simulink model "bee2_calib_corr"  (Simulink 7.1)
settings: Solver=ode45  StartTime=0.0  StopTime=100  RelTol=1e-3
undefined symbols (not defined in the file or in its folder): BEE2_usr
libraries: xbsIndex_r4 x2291, quant_xcorr_lib x1792, xps_library x56, xbsReplacements_r4 x32, casper_library_misc x2
(part 15 of 30)

== system bee2_calib_corr/quant_cross_corr1/lag-4 ==
  in1_0 : Inport  OutDataType=sfix(16)  OutScaling=2^0
  in1_1 : Inport  Port=2  OutDataType=sfix(16)  OutScaling=2^0
  in1_2 : Inport  Port=3  OutDataType=sfix(16)  OutScaling=2^0
  in1_3 : Inport  Port=4  OutDataType=sfix(16)  OutScaling=2^0
  in1_4 : Inport  Port=5  OutDataType=sfix(16)  OutScaling=2^0
  in1_5 : Inport  Port=6  OutDataType=sfix(16)  OutScaling=2^0
  in1_6 : Inport  Port=7  OutDataType=sfix(16)  OutScaling=2^0
  in1_7 : Inport  Port=8  OutDataType=sfix(16)  OutScaling=2^0
  in2_0 : Inport  Port=9  OutDataType=sfix(16)  OutScaling=2^0
  in2_1 : Inport  Port=10  OutDataType=sfix(16)  OutScaling=2^0
  in2_2 : Inport  Port=11  OutDataType=sfix(16)  OutScaling=2^0
  in2_3 : Inport  Port=12  OutDataType=sfix(16)  OutScaling=2^0
  in2_4 : Inport  Port=13  OutDataType=sfix(16)  OutScaling=2^0
  in2_5 : Inport  Port=14  OutDataType=sfix(16)  OutScaling=2^0
  in2_6 : Inport  Port=15  OutDataType=sfix(16)  OutScaling=2^0
  in2_7 : Inport  Port=16  OutDataType=sfix(16)  OutScaling=2^0
  rst : Inport  Port=17  OutDataType=sfix(16)  OutScaling=2^0
  en : Inport  Port=18  OutDataType=sfix(16)  OutScaling=2^0
  accumul-4 : xbsIndex_r4/Accumulator  operation=Add  n_bits=32  overflow=Saturate  scale=1  rst=on  hasbypass=off  en=on  dbl_ovrd=off  use_behavioral_HDL=on  implementation=Fabric  block_type=accum
      settings: infoedit="Adder or subtractor-based accumulator.   Output type and binary point position match the input.<P><P>Hardware notes: When \"Reinitialize with input 'b' on reset\" is selected, the accumulator is forced to run at the system rate even if the input 'b' is running at a slower rate."  xl_area=[0, 0, 0, 0, 0, 0, 0]  block_version=10.1.3
  add1_{1..4} (4 alike) : xbsIndex_r4/AddSub  mode=Addition  use_carryin=off  use_carryout=off  en=off  latency=1  precision=Full  arith_type=Unsigned  n_bits=8  bin_pt=2  quantization=Truncate  overflow=Wrap  dbl_ovrd=off  use_behavioral_HDL=on  pipelined=on  use_rpm=on  hw_selection=Fabric  block_type=addsub
      settings: xl_area=[0, 0, 0, 0, 0, 0, 0]  block_version=10.1.3
  add2_1 : xbsIndex_r4/AddSub  mode=Addition  use_carryin=off  use_carryout=off  en=off  latency=1  precision=Full  arith_type=Unsigned  n_bits=8  bin_pt=2  quantization=Truncate  overflow=Wrap  dbl_ovrd=off  use_behavioral_HDL=on  pipelined=on  use_rpm=on  hw_selection=Fabric  block_type=addsub
      settings: xl_area=[0, 0, 0, 0, 0, 0, 0]  block_version=10.1.3
  add2_2 : xbsIndex_r4/AddSub  mode=Addition  use_carryin=off  use_carryout=off  en=off  latency=1  precision=Full  arith_type=Unsigned  n_bits=8  bin_pt=2  quantization=Truncate  overflow=Wrap  dbl_ovrd=off  use_behavioral_HDL=on  pipelined=on  use_rpm=on  hw_selection=Fabric  block_type=addsub
      settings: xl_area=[0, 0, 0, 0, 0, 0, 0]  block_version=10.1.3
  add3_1 : xbsIndex_r4/AddSub  mode=Addition  use_carryin=off  use_carryout=off  en=off  latency=1  precision=Full  arith_type=Unsigned  n_bits=8  bin_pt=2  quantization=Truncate  overflow=Wrap  dbl_ovrd=off  use_behavioral_HDL=on  pipelined=on  use_rpm=on  hw_selection=Fabric  block_type=addsub
      settings: xl_area=[0, 0, 0, 0, 0, 0, 0]  block_version=10.1.3
  mult{1..8} (8 alike) : quant_xcorr_lib/2bit_mult  SystemSampleTime=-1
  acc_out : Outport  OutDataType=sfix(16)  OutScaling=2^0
  wires:
  in1_0/1 -> mult1/1
  in2_0/1 -> mult1/2
  in1_1/1 -> mult2/1
  in2_1/1 -> mult2/2
  in1_2/1 -> mult3/1
  in2_2/1 -> mult3/2
  in1_3/1 -> mult4/1
  in2_3/1 -> mult4/2
  in1_4/1 -> mult5/1
  in2_4/1 -> mult5/2
  in1_5/1 -> mult6/1
  in2_5/1 -> mult6/2
  in1_6/1 -> mult7/1
  in2_6/1 -> mult7/2
  in1_7/1 -> mult8/1
  in2_7/1 -> mult8/2
  mult1/1 -> add1_1/1
  mult2/1 -> add1_1/2
  mult3/1 -> add1_2/1
  mult4/1 -> add1_2/2
  mult5/1 -> add1_3/1
  mult6/1 -> add1_3/2
  mult7/1 -> add1_4/1
  mult8/1 -> add1_4/2
  add1_1/1 -> add2_1/1
  add1_2/1 -> add2_1/2
  add1_3/1 -> add2_2/1
  add1_4/1 -> add2_2/2
  add2_1/1 -> add3_1/1
  add2_2/1 -> add3_1/2
  add3_1/1 -> accumul-4/1
  accumul-4/1 -> acc_out/1
  rst/1 -> accumul-4/2
  en/1 -> accumul-4/3

== system bee2_calib_corr/quant_cross_corr1/lag-5 ==
  in1_0 : Inport  OutDataType=sfix(16)  OutScaling=2^0
  in1_1 : Inport  Port=2  OutDataType=sfix(16)  OutScaling=2^0
  in1_2 : Inport  Port=3  OutDataType=sfix(16)  OutScaling=2^0
  in1_3 : Inport  Port=4  OutDataType=sfix(16)  OutScaling=2^0
  in1_4 : Inport  Port=5  OutDataType=sfix(16)  OutScaling=2^0
  in1_5 : Inport  Port=6  OutDataType=sfix(16)  OutScaling=2^0
  in1_6 : Inport  Port=7  OutDataType=sfix(16)  OutScaling=2^0
  in1_7 : Inport  Port=8  OutDataType=sfix(16)  OutScaling=2^0
  in2_0 : Inport  Port=9  OutDataType=sfix(16)  OutScaling=2^0
  in2_1 : Inport  Port=10  OutDataType=sfix(16)  OutScaling=2^0
  in2_2 : Inport  Port=11  OutDataType=sfix(16)  OutScaling=2^0
  in2_3 : Inport  Port=12  OutDataType=sfix(16)  OutScaling=2^0
  in2_4 : Inport  Port=13  OutDataType=sfix(16)  OutScaling=2^0
  in2_5 : Inport  Port=14  OutDataType=sfix(16)  OutScaling=2^0
  in2_6 : Inport  Port=15  OutDataType=sfix(16)  OutScaling=2^0
  in2_7 : Inport  Port=16  OutDataType=sfix(16)  OutScaling=2^0
  rst : Inport  Port=17  OutDataType=sfix(16)  OutScaling=2^0
  en : Inport  Port=18  OutDataType=sfix(16)  OutScaling=2^0
  accumul-5 : xbsIndex_r4/Accumulator  operation=Add  n_bits=32  overflow=Saturate  scale=1  rst=on  hasbypass=off  en=on  dbl_ovrd=off  use_behavioral_HDL=on  implementation=Fabric  block_type=accum
      settings: infoedit="Adder or subtractor-based accumulator.   Output type and binary point position match the input.<P><P>Hardware notes: When \"Reinitialize with input 'b' on reset\" is selected, the accumulator is forced to run at the system rate even if the input 'b' is running at a slower rate."  xl_area=[0, 0, 0, 0, 0, 0, 0]  block_version=10.1.3
  add1_{1..4} (4 alike) : xbsIndex_r4/AddSub  mode=Addition  use_carryin=off  use_carryout=off  en=off  latency=1  precision=Full  arith_type=Unsigned  n_bits=8  bin_pt=2  quantization=Truncate  overflow=Wrap  dbl_ovrd=off  use_behavioral_HDL=on  pipelined=on  use_rpm=on  hw_selection=Fabric  block_type=addsub
      settings: xl_area=[0, 0, 0, 0, 0, 0, 0]  block_version=10.1.3
  add2_1 : xbsIndex_r4/AddSub  mode=Addition  use_carryin=off  use_carryout=off  en=off  latency=1  precision=Full  arith_type=Unsigned  n_bits=8  bin_pt=2  quantization=Truncate  overflow=Wrap  dbl_ovrd=off  use_behavioral_HDL=on  pipelined=on  use_rpm=on  hw_selection=Fabric  block_type=addsub
      settings: xl_area=[0, 0, 0, 0, 0, 0, 0]  block_version=10.1.3
  add2_2 : xbsIndex_r4/AddSub  mode=Addition  use_carryin=off  use_carryout=off  en=off  latency=1  precision=Full  arith_type=Unsigned  n_bits=8  bin_pt=2  quantization=Truncate  overflow=Wrap  dbl_ovrd=off  use_behavioral_HDL=on  pipelined=on  use_rpm=on  hw_selection=Fabric  block_type=addsub
      settings: xl_area=[0, 0, 0, 0, 0, 0, 0]  block_version=10.1.3
  add3_1 : xbsIndex_r4/AddSub  mode=Addition  use_carryin=off  use_carryout=off  en=off  latency=1  precision=Full  arith_type=Unsigned  n_bits=8  bin_pt=2  quantization=Truncate  overflow=Wrap  dbl_ovrd=off  use_behavioral_HDL=on  pipelined=on  use_rpm=on  hw_selection=Fabric  block_type=addsub
      settings: xl_area=[0, 0, 0, 0, 0, 0, 0]  block_version=10.1.3
  mult{1..8} (8 alike) : quant_xcorr_lib/2bit_mult  SystemSampleTime=-1
  acc_out : Outport  OutDataType=sfix(16)  OutScaling=2^0
  wires:
  in1_0/1 -> mult1/1
  in2_0/1 -> mult1/2
  in1_1/1 -> mult2/1
  in2_1/1 -> mult2/2
  in1_2/1 -> mult3/1
  in2_2/1 -> mult3/2
  in1_3/1 -> mult4/1
  in2_3/1 -> mult4/2
  in1_4/1 -> mult5/1
  in2_4/1 -> mult5/2
  in1_5/1 -> mult6/1
  in2_5/1 -> mult6/2
  in1_6/1 -> mult7/1
  in2_6/1 -> mult7/2
  in1_7/1 -> mult8/1
  in2_7/1 -> mult8/2
  mult1/1 -> add1_1/1
  mult2/1 -> add1_1/2
  mult3/1 -> add1_2/1
  mult4/1 -> add1_2/2
  mult5/1 -> add1_3/1
  mult6/1 -> add1_3/2
  mult7/1 -> add1_4/1
  mult8/1 -> add1_4/2
  add1_1/1 -> add2_1/1
  add1_2/1 -> add2_1/2
  add1_3/1 -> add2_2/1
  add1_4/1 -> add2_2/2
  add2_1/1 -> add3_1/1
  add2_2/1 -> add3_1/2
  add3_1/1 -> accumul-5/1
  accumul-5/1 -> acc_out/1
  rst/1 -> accumul-5/2
  en/1 -> accumul-5/3

== system bee2_calib_corr/quant_cross_corr1/lag-6 ==
  in1_0 : Inport  OutDataType=sfix(16)  OutScaling=2^0
  in1_1 : Inport  Port=2  OutDataType=sfix(16)  OutScaling=2^0
  in1_2 : Inport  Port=3  OutDataType=sfix(16)  OutScaling=2^0
  in1_3 : Inport  Port=4  OutDataType=sfix(16)  OutScaling=2^0
  in1_4 : Inport  Port=5  OutDataType=sfix(16)  OutScaling=2^0
  in1_5 : Inport  Port=6  OutDataType=sfix(16)  OutScaling=2^0
  in1_6 : Inport  Port=7  OutDataType=sfix(16)  OutScaling=2^0
  in1_7 : Inport  Port=8  OutDataType=sfix(16)  OutScaling=2^0
  in2_0 : Inport  Port=9  OutDataType=sfix(16)  OutScaling=2^0
  in2_1 : Inport  Port=10  OutDataType=sfix(16)  OutScaling=2^0
  in2_2 : Inport  Port=11  OutDataType=sfix(16)  OutScaling=2^0
  in2_3 : Inport  Port=12  OutDataType=sfix(16)  OutScaling=2^0
  in2_4 : Inport  Port=13  OutDataType=sfix(16)  OutScaling=2^0
  in2_5 : Inport  Port=14  OutDataType=sfix(16)  OutScaling=2^0
  in2_6 : Inport  Port=15  OutDataType=sfix(16)  OutScaling=2^0
  in2_7 : Inport  Port=16  OutDataType=sfix(16)  OutScaling=2^0
  rst : Inport  Port=17  OutDataType=sfix(16)  OutScaling=2^0
  en : Inport  Port=18  OutDataType=sfix(16)  OutScaling=2^0
  accumul-6 : xbsIndex_r4/Accumulator  operation=Add  n_bits=32  overflow=Saturate  scale=1  rst=on  hasbypass=off  en=on  dbl_ovrd=off  use_behavioral_HDL=on  implementation=Fabric  block_type=accum
      settings: infoedit="Adder or subtractor-based accumulator.   Output type and binary point position match the input.<P><P>Hardware notes: When \"Reinitialize with input 'b' on reset\" is selected, the accumulator is forced to run at the system rate even if the input 'b' is running at a slower rate."  xl_area=[0, 0, 0, 0, 0, 0, 0]  block_version=10.1.3
  add1_{1..4} (4 alike) : xbsIndex_r4/AddSub  mode=Addition  use_carryin=off  use_carryout=off  en=off  latency=1  precision=Full  arith_type=Unsigned  n_bits=8  bin_pt=2  quantization=Truncate  overflow=Wrap  dbl_ovrd=off  use_behavioral_HDL=on  pipelined=on  use_rpm=on  hw_selection=Fabric  block_type=addsub
      settings: xl_area=[0, 0, 0, 0, 0, 0, 0]  block_version=10.1.3
  add2_1 : xbsIndex_r4/AddSub  mode=Addition  use_carryin=off  use_carryout=off  en=off  latency=1  precision=Full  arith_type=Unsigned  n_bits=8  bin_pt=2  quantization=Truncate  overflow=Wrap  dbl_ovrd=off  use_behavioral_HDL=on  pipelined=on  use_rpm=on  hw_selection=Fabric  block_type=addsub
      settings: xl_area=[0, 0, 0, 0, 0, 0, 0]  block_version=10.1.3
  add2_2 : xbsIndex_r4/AddSub  mode=Addition  use_carryin=off  use_carryout=off  en=off  latency=1  precision=Full  arith_type=Unsigned  n_bits=8  bin_pt=2  quantization=Truncate  overflow=Wrap  dbl_ovrd=off  use_behavioral_HDL=on  pipelined=on  use_rpm=on  hw_selection=Fabric  block_type=addsub
      settings: xl_area=[0, 0, 0, 0, 0, 0, 0]  block_version=10.1.3
  add3_1 : xbsIndex_r4/AddSub  mode=Addition  use_carryin=off  use_carryout=off  en=off  latency=1  precision=Full  arith_type=Unsigned  n_bits=8  bin_pt=2  quantization=Truncate  overflow=Wrap  dbl_ovrd=off  use_behavioral_HDL=on  pipelined=on  use_rpm=on  hw_selection=Fabric  block_type=addsub
      settings: xl_area=[0, 0, 0, 0, 0, 0, 0]  block_version=10.1.3
  mult{1..8} (8 alike) : quant_xcorr_lib/2bit_mult  SystemSampleTime=-1
  acc_out : Outport  OutDataType=sfix(16)  OutScaling=2^0
  wires:
  in1_0/1 -> mult1/1
  in2_0/1 -> mult1/2
  in1_1/1 -> mult2/1
  in2_1/1 -> mult2/2
  in1_2/1 -> mult3/1
  in2_2/1 -> mult3/2
  in1_3/1 -> mult4/1
  in2_3/1 -> mult4/2
  in1_4/1 -> mult5/1
  in2_4/1 -> mult5/2
  in1_5/1 -> mult6/1
  in2_5/1 -> mult6/2
  in1_6/1 -> mult7/1
  in2_6/1 -> mult7/2
  in1_7/1 -> mult8/1
  in2_7/1 -> mult8/2
  mult1/1 -> add1_1/1
  mult2/1 -> add1_1/2
  mult3/1 -> add1_2/1
  mult4/1 -> add1_2/2
  mult5/1 -> add1_3/1
  mult6/1 -> add1_3/2
  mult7/1 -> add1_4/1
  mult8/1 -> add1_4/2
  add1_1/1 -> add2_1/1
  add1_2/1 -> add2_1/2
  add1_3/1 -> add2_2/1
  add1_4/1 -> add2_2/2
  add2_1/1 -> add3_1/1
  add2_2/1 -> add3_1/2
  add3_1/1 -> accumul-6/1
  accumul-6/1 -> acc_out/1
  rst/1 -> accumul-6/2
  en/1 -> accumul-6/3

== system bee2_calib_corr/quant_cross_corr1/lag-7 ==
  in1_0 : Inport  OutDataType=sfix(16)  OutScaling=2^0
  in1_1 : Inport  Port=2  OutDataType=sfix(16)  OutScaling=2^0
  in1_2 : Inport  Port=3  OutDataType=sfix(16)  OutScaling=2^0
  in1_3 : Inport  Port=4  OutDataType=sfix(16)  OutScaling=2^0
  in1_4 : Inport  Port=5  OutDataType=sfix(16)  OutScaling=2^0
  in1_5 : Inport  Port=6  OutDataType=sfix(16)  OutScaling=2^0
  in1_6 : Inport  Port=7  OutDataType=sfix(16)  OutScaling=2^0
  in1_7 : Inport  Port=8  OutDataType=sfix(16)  OutScaling=2^0
  in2_0 : Inport  Port=9  OutDataType=sfix(16)  OutScaling=2^0
  in2_1 : Inport  Port=10  OutDataType=sfix(16)  OutScaling=2^0
  in2_2 : Inport  Port=11  OutDataType=sfix(16)  OutScaling=2^0
  in2_3 : Inport  Port=12  OutDataType=sfix(16)  OutScaling=2^0
  in2_4 : Inport  Port=13  OutDataType=sfix(16)  OutScaling=2^0
  in2_5 : Inport  Port=14  OutDataType=sfix(16)  OutScaling=2^0
  in2_6 : Inport  Port=15  OutDataType=sfix(16)  OutScaling=2^0
  in2_7 : Inport  Port=16  OutDataType=sfix(16)  OutScaling=2^0
  rst : Inport  Port=17  OutDataType=sfix(16)  OutScaling=2^0
  en : Inport  Port=18  OutDataType=sfix(16)  OutScaling=2^0
  accumul-7 : xbsIndex_r4/Accumulator  operation=Add  n_bits=32  overflow=Saturate  scale=1  rst=on  hasbypass=off  en=on  dbl_ovrd=off  use_behavioral_HDL=on  implementation=Fabric  block_type=accum
      settings: infoedit="Adder or subtractor-based accumulator.   Output type and binary point position match the input.<P><P>Hardware notes: When \"Reinitialize with input 'b' on reset\" is selected, the accumulator is forced to run at the system rate even if the input 'b' is running at a slower rate."  xl_area=[0, 0, 0, 0, 0, 0, 0]  block_version=10.1.3
  add1_{1..4} (4 alike) : xbsIndex_r4/AddSub  mode=Addition  use_carryin=off  use_carryout=off  en=off  latency=1  precision=Full  arith_type=Unsigned  n_bits=8  bin_pt=2  quantization=Truncate  overflow=Wrap  dbl_ovrd=off  use_behavioral_HDL=on  pipelined=on  use_rpm=on  hw_selection=Fabric  block_type=addsub
      settings: xl_area=[0, 0, 0, 0, 0, 0, 0]  block_version=10.1.3
  add2_1 : xbsIndex_r4/AddSub  mode=Addition  use_carryin=off  use_carryout=off  en=off  latency=1  precision=Full  arith_type=Unsigned  n_bits=8  bin_pt=2  quantization=Truncate  overflow=Wrap  dbl_ovrd=off  use_behavioral_HDL=on  pipelined=on  use_rpm=on  hw_selection=Fabric  block_type=addsub
      settings: xl_area=[0, 0, 0, 0, 0, 0, 0]  block_version=10.1.3
  add2_2 : xbsIndex_r4/AddSub  mode=Addition  use_carryin=off  use_carryout=off  en=off  latency=1  precision=Full  arith_type=Unsigned  n_bits=8  bin_pt=2  quantization=Truncate  overflow=Wrap  dbl_ovrd=off  use_behavioral_HDL=on  pipelined=on  use_rpm=on  hw_selection=Fabric  block_type=addsub
      settings: xl_area=[0, 0, 0, 0, 0, 0, 0]  block_version=10.1.3
  add3_1 : xbsIndex_r4/AddSub  mode=Addition  use_carryin=off  use_carryout=off  en=off  latency=1  precision=Full  arith_type=Unsigned  n_bits=8  bin_pt=2  quantization=Truncate  overflow=Wrap  dbl_ovrd=off  use_behavioral_HDL=on  pipelined=on  use_rpm=on  hw_selection=Fabric  block_type=addsub
      settings: xl_area=[0, 0, 0, 0, 0, 0, 0]  block_version=10.1.3
  mult{1..8} (8 alike) : quant_xcorr_lib/2bit_mult  SystemSampleTime=-1
  acc_out : Outport  OutDataType=sfix(16)  OutScaling=2^0
  wires:
  in1_0/1 -> mult1/1
  in2_0/1 -> mult1/2
  in1_1/1 -> mult2/1
  in2_1/1 -> mult2/2
  in1_2/1 -> mult3/1
  in2_2/1 -> mult3/2
  in1_3/1 -> mult4/1
  in2_3/1 -> mult4/2
  in1_4/1 -> mult5/1
  in2_4/1 -> mult5/2
  in1_5/1 -> mult6/1
  in2_5/1 -> mult6/2
  in1_6/1 -> mult7/1
  in2_6/1 -> mult7/2
  in1_7/1 -> mult8/1
  in2_7/1 -> mult8/2
  mult1/1 -> add1_1/1
  mult2/1 -> add1_1/2
  mult3/1 -> add1_2/1
  mult4/1 -> add1_2/2
  mult5/1 -> add1_3/1
  mult6/1 -> add1_3/2
  mult7/1 -> add1_4/1
  mult8/1 -> add1_4/2
  add1_1/1 -> add2_1/1
  add1_2/1 -> add2_1/2
  add1_3/1 -> add2_2/1
  add1_4/1 -> add2_2/2
  add2_1/1 -> add3_1/1
  add2_2/1 -> add3_1/2
  add3_1/1 -> accumul-7/1
  accumul-7/1 -> acc_out/1
  rst/1 -> accumul-7/2
  en/1 -> accumul-7/3

== system bee2_calib_corr/quant_cross_corr1/lag-8 ==
  in1_0 : Inport  OutDataType=sfix(16)  OutScaling=2^0
  in1_1 : Inport  Port=2  OutDataType=sfix(16)  OutScaling=2^0
  in1_2 : Inport  Port=3  OutDataType=sfix(16)  OutScaling=2^0
  in1_3 : Inport  Port=4  OutDataType=sfix(16)  OutScaling=2^0
  in1_4 : Inport  Port=5  OutDataType=sfix(16)  OutScaling=2^0
  in1_5 : Inport  Port=6  OutDataType=sfix(16)  OutScaling=2^0
  in1_6 : Inport  Port=7  OutDataType=sfix(16)  OutScaling=2^0
  in1_7 : Inport  Port=8  OutDataType=sfix(16)  OutScaling=2^0
  in2_0 : Inport  Port=9  OutDataType=sfix(16)  OutScaling=2^0
  in2_1 : Inport  Port=10  OutDataType=sfix(16)  OutScaling=2^0
  in2_2 : Inport  Port=11  OutDataType=sfix(16)  OutScaling=2^0
  in2_3 : Inport  Port=12  OutDataType=sfix(16)  OutScaling=2^0
  in2_4 : Inport  Port=13  OutDataType=sfix(16)  OutScaling=2^0
  in2_5 : Inport  Port=14  OutDataType=sfix(16)  OutScaling=2^0
  in2_6 : Inport  Port=15  OutDataType=sfix(16)  OutScaling=2^0
  in2_7 : Inport  Port=16  OutDataType=sfix(16)  OutScaling=2^0
  rst : Inport  Port=17  OutDataType=sfix(16)  OutScaling=2^0
  en : Inport  Port=18  OutDataType=sfix(16)  OutScaling=2^0
  accumul-8 : xbsIndex_r4/Accumulator  operation=Add  n_bits=32  overflow=Saturate  scale=1  rst=on  hasbypass=off  en=on  dbl_ovrd=off  use_behavioral_HDL=on  implementation=Fabric  block_type=accum
      settings: infoedit="Adder or subtractor-based accumulator.   Output type and binary point position match the input.<P><P>Hardware notes: When \"Reinitialize with input 'b' on reset\" is selected, the accumulator is forced to run at the system rate even if the input 'b' is running at a slower rate."  xl_area=[0, 0, 0, 0, 0, 0, 0]  block_version=10.1.3
  add1_{1..4} (4 alike) : xbsIndex_r4/AddSub  mode=Addition  use_carryin=off  use_carryout=off  en=off  latency=1  precision=Full  arith_type=Unsigned  n_bits=8  bin_pt=2  quantization=Truncate  overflow=Wrap  dbl_ovrd=off  use_behavioral_HDL=on  pipelined=on  use_rpm=on  hw_selection=Fabric  block_type=addsub
      settings: xl_area=[0, 0, 0, 0, 0, 0, 0]  block_version=10.1.3
  add2_1 : xbsIndex_r4/AddSub  mode=Addition  use_carryin=off  use_carryout=off  en=off  latency=1  precision=Full  arith_type=Unsigned  n_bits=8  bin_pt=2  quantization=Truncate  overflow=Wrap  dbl_ovrd=off  use_behavioral_HDL=on  pipelined=on  use_rpm=on  hw_selection=Fabric  block_type=addsub
      settings: xl_area=[0, 0, 0, 0, 0, 0, 0]  block_version=10.1.3
  add2_2 : xbsIndex_r4/AddSub  mode=Addition  use_carryin=off  use_carryout=off  en=off  latency=1  precision=Full  arith_type=Unsigned  n_bits=8  bin_pt=2  quantization=Truncate  overflow=Wrap  dbl_ovrd=off  use_behavioral_HDL=on  pipelined=on  use_rpm=on  hw_selection=Fabric  block_type=addsub
      settings: xl_area=[0, 0, 0, 0, 0, 0, 0]  block_version=10.1.3
  add3_1 : xbsIndex_r4/AddSub  mode=Addition  use_carryin=off  use_carryout=off  en=off  latency=1  precision=Full  arith_type=Unsigned  n_bits=8  bin_pt=2  quantization=Truncate  overflow=Wrap  dbl_ovrd=off  use_behavioral_HDL=on  pipelined=on  use_rpm=on  hw_selection=Fabric  block_type=addsub
      settings: xl_area=[0, 0, 0, 0, 0, 0, 0]  block_version=10.1.3
  mult{1..8} (8 alike) : quant_xcorr_lib/2bit_mult  SystemSampleTime=-1
  acc_out : Outport  OutDataType=sfix(16)  OutScaling=2^0
  wires:
  in1_0/1 -> mult1/1
  in2_0/1 -> mult1/2
  in1_1/1 -> mult2/1
  in2_1/1 -> mult2/2
  in1_2/1 -> mult3/1
  in2_2/1 -> mult3/2
  in1_3/1 -> mult4/1
  in2_3/1 -> mult4/2
  in1_4/1 -> mult5/1
  in2_4/1 -> mult5/2
  in1_5/1 -> mult6/1
  in2_5/1 -> mult6/2
  in1_6/1 -> mult7/1
  in2_6/1 -> mult7/2
  in1_7/1 -> mult8/1
  in2_7/1 -> mult8/2
  mult1/1 -> add1_1/1
  mult2/1 -> add1_1/2
  mult3/1 -> add1_2/1
  mult4/1 -> add1_2/2
  mult5/1 -> add1_3/1
  mult6/1 -> add1_3/2
  mult7/1 -> add1_4/1
  mult8/1 -> add1_4/2
  add1_1/1 -> add2_1/1
  add1_2/1 -> add2_1/2
  add1_3/1 -> add2_2/1
  add1_4/1 -> add2_2/2
  add2_1/1 -> add3_1/1
  add2_2/1 -> add3_1/2
  add3_1/1 -> accumul-8/1
  accumul-8/1 -> acc_out/1
  rst/1 -> accumul-8/2
  en/1 -> accumul-8/3

== system bee2_calib_corr/quant_cross_corr1/lag-9 ==
  in1_0 : Inport  OutDataType=sfix(16)  OutScaling=2^0
  in1_1 : Inport  Port=2  OutDataType=sfix(16)  OutScaling=2^0
  in1_2 : Inport  Port=3  OutDataType=sfix(16)  OutScaling=2^0
  in1_3 : Inport  Port=4  OutDataType=sfix(16)  OutScaling=2^0
  in1_4 : Inport  Port=5  OutDataType=sfix(16)  OutScaling=2^0
  in1_5 : Inport  Port=6  OutDataType=sfix(16)  OutScaling=2^0
  in1_6 : Inport  Port=7  OutDataType=sfix(16)  OutScaling=2^0
  in1_7 : Inport  Port=8  OutDataType=sfix(16)  OutScaling=2^0
  in2_0 : Inport  Port=9  OutDataType=sfix(16)  OutScaling=2^0
  in2_1 : Inport  Port=10  OutDataType=sfix(16)  OutScaling=2^0
  in2_2 : Inport  Port=11  OutDataType=sfix(16)  OutScaling=2^0
  in2_3 : Inport  Port=12  OutDataType=sfix(16)  OutScaling=2^0
  in2_4 : Inport  Port=13  OutDataType=sfix(16)  OutScaling=2^0
  in2_5 : Inport  Port=14  OutDataType=sfix(16)  OutScaling=2^0
  in2_6 : Inport  Port=15  OutDataType=sfix(16)  OutScaling=2^0
  in2_7 : Inport  Port=16  OutDataType=sfix(16)  OutScaling=2^0
  rst : Inport  Port=17  OutDataType=sfix(16)  OutScaling=2^0
  en : Inport  Port=18  OutDataType=sfix(16)  OutScaling=2^0
  accumul-9 : xbsIndex_r4/Accumulator  operation=Add  n_bits=32  overflow=Saturate  scale=1  rst=on  hasbypass=off  en=on  dbl_ovrd=off  use_behavioral_HDL=on  implementation=Fabric  block_type=accum
      settings: infoedit="Adder or subtractor-based accumulator.   Output type and binary point position match the input.<P><P>Hardware notes: When \"Reinitialize with input 'b' on reset\" is selected, the accumulator is forced to run at the system rate even if the input 'b' is running at a slower rate."  xl_area=[0, 0, 0, 0, 0, 0, 0]  block_version=10.1.3
  add1_{1..4} (4 alike) : xbsIndex_r4/AddSub  mode=Addition  use_carryin=off  use_carryout=off  en=off  latency=1  precision=Full  arith_type=Unsigned  n_bits=8  bin_pt=2  quantization=Truncate  overflow=Wrap  dbl_ovrd=off  use_behavioral_HDL=on  pipelined=on  use_rpm=on  hw_selection=Fabric  block_type=addsub
      settings: xl_area=[0, 0, 0, 0, 0, 0, 0]  block_version=10.1.3
  add2_1 : xbsIndex_r4/AddSub  mode=Addition  use_carryin=off  use_carryout=off  en=off  latency=1  precision=Full  arith_type=Unsigned  n_bits=8  bin_pt=2  quantization=Truncate  overflow=Wrap  dbl_ovrd=off  use_behavioral_HDL=on  pipelined=on  use_rpm=on  hw_selection=Fabric  block_type=addsub
      settings: xl_area=[0, 0, 0, 0, 0, 0, 0]  block_version=10.1.3
  add2_2 : xbsIndex_r4/AddSub  mode=Addition  use_carryin=off  use_carryout=off  en=off  latency=1  precision=Full  arith_type=Unsigned  n_bits=8  bin_pt=2  quantization=Truncate  overflow=Wrap  dbl_ovrd=off  use_behavioral_HDL=on  pipelined=on  use_rpm=on  hw_selection=Fabric  block_type=addsub
      settings: xl_area=[0, 0, 0, 0, 0, 0, 0]  block_version=10.1.3
  add3_1 : xbsIndex_r4/AddSub  mode=Addition  use_carryin=off  use_carryout=off  en=off  latency=1  precision=Full  arith_type=Unsigned  n_bits=8  bin_pt=2  quantization=Truncate  overflow=Wrap  dbl_ovrd=off  use_behavioral_HDL=on  pipelined=on  use_rpm=on  hw_selection=Fabric  block_type=addsub
      settings: xl_area=[0, 0, 0, 0, 0, 0, 0]  block_version=10.1.3
  mult{1..8} (8 alike) : quant_xcorr_lib/2bit_mult  SystemSampleTime=-1
  acc_out : Outport  OutDataType=sfix(16)  OutScaling=2^0
  wires:
  in1_0/1 -> mult1/1
  in2_0/1 -> mult1/2
  in1_1/1 -> mult2/1
  in2_1/1 -> mult2/2
  in1_2/1 -> mult3/1
  in2_2/1 -> mult3/2
  in1_3/1 -> mult4/1
  in2_3/1 -> mult4/2
  in1_4/1 -> mult5/1
  in2_4/1 -> mult5/2
  in1_5/1 -> mult6/1
  in2_5/1 -> mult6/2
  in1_6/1 -> mult7/1
  in2_6/1 -> mult7/2
  in1_7/1 -> mult8/1
  in2_7/1 -> mult8/2
  mult1/1 -> add1_1/1
  mult2/1 -> add1_1/2
  mult3/1 -> add1_2/1
  mult4/1 -> add1_2/2
  mult5/1 -> add1_3/1
  mult6/1 -> add1_3/2
  mult7/1 -> add1_4/1
  mult8/1 -> add1_4/2
  add1_1/1 -> add2_1/1
  add1_2/1 -> add2_1/2
  add1_3/1 -> add2_2/1
  add1_4/1 -> add2_2/2
  add2_1/1 -> add3_1/1
  add2_2/1 -> add3_1/2
  add3_1/1 -> accumul-9/1
  accumul-9/1 -> acc_out/1
  rst/1 -> accumul-9/2
  en/1 -> accumul-9/3

== system bee2_calib_corr/quant_cross_corr1/lag0 ==
  in1_0 : Inport  OutDataType=sfix(16)  OutScaling=2^0
  in1_1 : Inport  Port=2  OutDataType=sfix(16)  OutScaling=2^0
  in1_2 : Inport  Port=3  OutDataType=sfix(16)  OutScaling=2^0
  in1_3 : Inport  Port=4  OutDataType=sfix(16)  OutScaling=2^0
  in1_4 : Inport  Port=5  OutDataType=sfix(16)  OutScaling=2^0
  in1_5 : Inport  Port=6  OutDataType=sfix(16)  OutScaling=2^0
  in1_6 : Inport  Port=7  OutDataType=sfix(16)  OutScaling=2^0
  in1_7 : Inport  Port=8  OutDataType=sfix(16)  OutScaling=2^0
  in2_0 : Inport  Port=9  OutDataType=sfix(16)  OutScaling=2^0
  in2_1 : Inport  Port=10  OutDataType=sfix(16)  OutScaling=2^0
  in2_2 : Inport  Port=11  OutDataType=sfix(16)  OutScaling=2^0
  in2_3 : Inport  Port=12  OutDataType=sfix(16)  OutScaling=2^0
  in2_4 : Inport  Port=13  OutDataType=sfix(16)  OutScaling=2^0
  in2_5 : Inport  Port=14  OutDataType=sfix(16)  OutScaling=2^0
  in2_6 : Inport  Port=15  OutDataType=sfix(16)  OutScaling=2^0
  in2_7 : Inport  Port=16  OutDataType=sfix(16)  OutScaling=2^0
  rst : Inport  Port=17  OutDataType=sfix(16)  OutScaling=2^0
  en : Inport  Port=18  OutDataType=sfix(16)  OutScaling=2^0
  accumul0 : xbsIndex_r4/Accumulator  operation=Add  n_bits=32  overflow=Saturate  scale=1  rst=on  hasbypass=off  en=on  dbl_ovrd=off  use_behavioral_HDL=on  implementation=Fabric  block_type=accum
      settings: infoedit="Adder or subtractor-based accumulator.   Output type and binary point position match the input.<P><P>Hardware notes: When \"Reinitialize with input 'b' on reset\" is selected, the accumulator is forced to run at the system rate even if the input 'b' is running at a slower rate."  xl_area=[0, 0, 0, 0, 0, 0, 0]  block_version=10.1.3
  add1_{1..4} (4 alike) : xbsIndex_r4/AddSub  mode=Addition  use_carryin=off  use_carryout=off  en=off  latency=1  precision=Full  arith_type=Unsigned  n_bits=8  bin_pt=2  quantization=Truncate  overflow=Wrap  dbl_ovrd=off  use_behavioral_HDL=on  pipelined=on  use_rpm=on  hw_selection=Fabric  block_type=addsub
      settings: xl_area=[0, 0, 0, 0, 0, 0, 0]  block_version=10.1.3
  add2_1 : xbsIndex_r4/AddSub  mode=Addition  use_carryin=off  use_carryout=off  en=off  latency=1  precision=Full  arith_type=Unsigned  n_bits=8  bin_pt=2  quantization=Truncate  overflow=Wrap  dbl_ovrd=off  use_behavioral_HDL=on  pipelined=on  use_rpm=on  hw_selection=Fabric  block_type=addsub
      settings: xl_area=[0, 0, 0, 0, 0, 0, 0]  block_version=10.1.3
  add2_2 : xbsIndex_r4/AddSub  mode=Addition  use_carryin=off  use_carryout=off  en=off  latency=1  precision=Full  arith_type=Unsigned  n_bits=8  bin_pt=2  quantization=Truncate  overflow=Wrap  dbl_ovrd=off  use_behavioral_HDL=on  pipelined=on  use_rpm=on  hw_selection=Fabric  block_type=addsub
      settings: xl_area=[0, 0, 0, 0, 0, 0, 0]  block_version=10.1.3
  add3_1 : xbsIndex_r4/AddSub  mode=Addition  use_carryin=off  use_carryout=off  en=off  latency=1  precision=Full  arith_type=Unsigned  n_bits=8  bin_pt=2  quantization=Truncate  overflow=Wrap  dbl_ovrd=off  use_behavioral_HDL=on  pipelined=on  use_rpm=on  hw_selection=Fabric  block_type=addsub
      settings: xl_area=[0, 0, 0, 0, 0, 0, 0]  block_version=10.1.3
  mult{1..8} (8 alike) : quant_xcorr_lib/2bit_mult  SystemSampleTime=-1
  acc_out : Outport  OutDataType=sfix(16)  OutScaling=2^0
  wires:
  in1_0/1 -> mult1/1
  in2_0/1 -> mult1/2
  in1_1/1 -> mult2/1
  in2_1/1 -> mult2/2
  in1_2/1 -> mult3/1
  in2_2/1 -> mult3/2
  in1_3/1 -> mult4/1
  in2_3/1 -> mult4/2
  in1_4/1 -> mult5/1
  in2_4/1 -> mult5/2
  in1_5/1 -> mult6/1
  in2_5/1 -> mult6/2
  in1_6/1 -> mult7/1
  in2_6/1 -> mult7/2
  in1_7/1 -> mult8/1
  in2_7/1 -> mult8/2
  mult1/1 -> add1_1/1
  mult2/1 -> add1_1/2
  mult3/1 -> add1_2/1
  mult4/1 -> add1_2/2
  mult5/1 -> add1_3/1
  mult6/1 -> add1_3/2
  mult7/1 -> add1_4/1
  mult8/1 -> add1_4/2
  add1_1/1 -> add2_1/1
  add1_2/1 -> add2_1/2
  add1_3/1 -> add2_2/1
  add1_4/1 -> add2_2/2
  add2_1/1 -> add3_1/1
  add2_2/1 -> add3_1/2
  add3_1/1 -> accumul0/1
  accumul0/1 -> acc_out/1
  rst/1 -> accumul0/2
  en/1 -> accumul0/3

== system bee2_calib_corr/quant_cross_corr1/lag1 ==
  in1_0 : Inport  OutDataType=sfix(16)  OutScaling=2^0
  in1_1 : Inport  Port=2  OutDataType=sfix(16)  OutScaling=2^0
  in1_2 : Inport  Port=3  OutDataType=sfix(16)  OutScaling=2^0
  in1_3 : Inport  Port=4  OutDataType=sfix(16)  OutScaling=2^0
  in1_4 : Inport  Port=5  OutDataType=sfix(16)  OutScaling=2^0
  in1_5 : Inport  Port=6  OutDataType=sfix(16)  OutScaling=2^0
  in1_6 : Inport  Port=7  OutDataType=sfix(16)  OutScaling=2^0
  in1_7 : Inport  Port=8  OutDataType=sfix(16)  OutScaling=2^0
  in2_0 : Inport  Port=9  OutDataType=sfix(16)  OutScaling=2^0
  in2_1 : Inport  Port=10  OutDataType=sfix(16)  OutScaling=2^0
  in2_2 : Inport  Port=11  OutDataType=sfix(16)  OutScaling=2^0
  in2_3 : Inport  Port=12  OutDataType=sfix(16)  OutScaling=2^0
  in2_4 : Inport  Port=13  OutDataType=sfix(16)  OutScaling=2^0
  in2_5 : Inport  Port=14  OutDataType=sfix(16)  OutScaling=2^0
  in2_6 : Inport  Port=15  OutDataType=sfix(16)  OutScaling=2^0
  in2_7 : Inport  Port=16  OutDataType=sfix(16)  OutScaling=2^0
  rst : Inport  Port=17  OutDataType=sfix(16)  OutScaling=2^0
  en : Inport  Port=18  OutDataType=sfix(16)  OutScaling=2^0
  accumul1 : xbsIndex_r4/Accumulator  operation=Add  n_bits=32  overflow=Saturate  scale=1  rst=on  hasbypass=off  en=on  dbl_ovrd=off  use_behavioral_HDL=on  implementation=Fabric  block_type=accum
      settings: infoedit="Adder or subtractor-based accumulator.   Output type and binary point position match the input.<P><P>Hardware notes: When \"Reinitialize with input 'b' on reset\" is selected, the accumulator is forced to run at the system rate even if the input 'b' is running at a slower rate."  xl_area=[0, 0, 0, 0, 0, 0, 0]  block_version=10.1.3
  add1_{1..4} (4 alike) : xbsIndex_r4/AddSub  mode=Addition  use_carryin=off  use_carryout=off  en=off  latency=1  precision=Full  arith_type=Unsigned  n_bits=8  bin_pt=2  quantization=Truncate  overflow=Wrap  dbl_ovrd=off  use_behavioral_HDL=on  pipelined=on  use_rpm=on  hw_selection=Fabric  block_type=addsub
      settings: xl_area=[0, 0, 0, 0, 0, 0, 0]  block_version=10.1.3
  add2_1 : xbsIndex_r4/AddSub  mode=Addition  use_carryin=off  use_carryout=off  en=off  latency=1  precision=Full  arith_type=Unsigned  n_bits=8  bin_pt=2  quantization=Truncate  overflow=Wrap  dbl_ovrd=off  use_behavioral_HDL=on  pipelined=on  use_rpm=on  hw_selection=Fabric  block_type=addsub
      settings: xl_area=[0, 0, 0, 0, 0, 0, 0]  block_version=10.1.3
  add2_2 : xbsIndex_r4/AddSub  mode=Addition  use_carryin=off  use_carryout=off  en=off  latency=1  precision=Full  arith_type=Unsigned  n_bits=8  bin_pt=2  quantization=Truncate  overflow=Wrap  dbl_ovrd=off  use_behavioral_HDL=on  pipelined=on  use_rpm=on  hw_selection=Fabric  block_type=addsub
      settings: xl_area=[0, 0, 0, 0, 0, 0, 0]  block_version=10.1.3
  add3_1 : xbsIndex_r4/AddSub  mode=Addition  use_carryin=off  use_carryout=off  en=off  latency=1  precision=Full  arith_type=Unsigned  n_bits=8  bin_pt=2  quantization=Truncate  overflow=Wrap  dbl_ovrd=off  use_behavioral_HDL=on  pipelined=on  use_rpm=on  hw_selection=Fabric  block_type=addsub
      settings: xl_area=[0, 0, 0, 0, 0, 0, 0]  block_version=10.1.3
  mult{1..8} (8 alike) : quant_xcorr_lib/2bit_mult  SystemSampleTime=-1
  acc_out : Outport  OutDataType=sfix(16)  OutScaling=2^0
  wires:
  in1_0/1 -> mult1/1
  in2_0/1 -> mult1/2
  in1_1/1 -> mult2/1
  in2_1/1 -> mult2/2
  in1_2/1 -> mult3/1
  in2_2/1 -> mult3/2
  in1_3/1 -> mult4/1
  in2_3/1 -> mult4/2
  in1_4/1 -> mult5/1
  in2_4/1 -> mult5/2
  in1_5/1 -> mult6/1
  in2_5/1 -> mult6/2
  in1_6/1 -> mult7/1
  in2_6/1 -> mult7/2
  in1_7/1 -> mult8/1
  in2_7/1 -> mult8/2
  mult1/1 -> add1_1/1
  mult2/1 -> add1_1/2
  mult3/1 -> add1_2/1
  mult4/1 -> add1_2/2
  mult5/1 -> add1_3/1
  mult6/1 -> add1_3/2
  mult7/1 -> add1_4/1
  mult8/1 -> add1_4/2
  add1_1/1 -> add2_1/1
  add1_2/1 -> add2_1/2
  add1_3/1 -> add2_2/1
  add1_4/1 -> add2_2/2
  add2_1/1 -> add3_1/1
  add2_2/1 -> add3_1/2
  add3_1/1 -> accumul1/1
  accumul1/1 -> acc_out/1
  rst/1 -> accumul1/2
  en/1 -> accumul1/3

== system bee2_calib_corr/quant_cross_corr1/lag10 ==
  in1_0 : Inport  OutDataType=sfix(16)  OutScaling=2^0
  in1_1 : Inport  Port=2  OutDataType=sfix(16)  OutScaling=2^0
  in1_2 : Inport  Port=3  OutDataType=sfix(16)  OutScaling=2^0
  in1_3 : Inport  Port=4  OutDataType=sfix(16)  OutScaling=2^0
  in1_4 : Inport  Port=5  OutDataType=sfix(16)  OutScaling=2^0
  in1_5 : Inport  Port=6  OutDataType=sfix(16)  OutScaling=2^0
  in1_6 : Inport  Port=7  OutDataType=sfix(16)  OutScaling=2^0
  in1_7 : Inport  Port=8  OutDataType=sfix(16)  OutScaling=2^0
  in2_0 : Inport  Port=9  OutDataType=sfix(16)  OutScaling=2^0
  in2_1 : Inport  Port=10  OutDataType=sfix(16)  OutScaling=2^0
  in2_2 : Inport  Port=11  OutDataType=sfix(16)  OutScaling=2^0
  in2_3 : Inport  Port=12  OutDataType=sfix(16)  OutScaling=2^0
  in2_4 : Inport  Port=13  OutDataType=sfix(16)  OutScaling=2^0
  in2_5 : Inport  Port=14  OutDataType=sfix(16)  OutScaling=2^0
  in2_6 : Inport  Port=15  OutDataType=sfix(16)  OutScaling=2^0
  in2_7 : Inport  Port=16  OutDataType=sfix(16)  OutScaling=2^0
  rst : Inport  Port=17  OutDataType=sfix(16)  OutScaling=2^0
  en : Inport  Port=18  OutDataType=sfix(16)  OutScaling=2^0
  accumul10 : xbsIndex_r4/Accumulator  operation=Add  n_bits=32  overflow=Saturate  scale=1  rst=on  hasbypass=off  en=on  dbl_ovrd=off  use_behavioral_HDL=on  implementation=Fabric  block_type=accum
      settings: infoedit="Adder or subtractor-based accumulator.   Output type and binary point position match the input.<P><P>Hardware notes: When \"Reinitialize with input 'b' on reset\" is selected, the accumulator is forced to run at the system rate even if the input 'b' is running at a slower rate."  xl_area=[0, 0, 0, 0, 0, 0, 0]  block_version=10.1.3
  add1_{1..4} (4 alike) : xbsIndex_r4/AddSub  mode=Addition  use_carryin=off  use_carryout=off  en=off  latency=1  precision=Full  arith_type=Unsigned  n_bits=8  bin_pt=2  quantization=Truncate  overflow=Wrap  dbl_ovrd=off  use_behavioral_HDL=on  pipelined=on  use_rpm=on  hw_selection=Fabric  block_type=addsub
      settings: xl_area=[0, 0, 0, 0, 0, 0, 0]  block_version=10.1.3
  add2_1 : xbsIndex_r4/AddSub  mode=Addition  use_carryin=off  use_carryout=off  en=off  latency=1  precision=Full  arith_type=Unsigned  n_bits=8  bin_pt=2  quantization=Truncate  overflow=Wrap  dbl_ovrd=off  use_behavioral_HDL=on  pipelined=on  use_rpm=on  hw_selection=Fabric  block_type=addsub
      settings: xl_area=[0, 0, 0, 0, 0, 0, 0]  block_version=10.1.3
  add2_2 : xbsIndex_r4/AddSub  mode=Addition  use_carryin=off  use_carryout=off  en=off  latency=1  precision=Full  arith_type=Unsigned  n_bits=8  bin_pt=2  quantization=Truncate  overflow=Wrap  dbl_ovrd=off  use_behavioral_HDL=on  pipelined=on  use_rpm=on  hw_selection=Fabric  block_type=addsub
      settings: xl_area=[0, 0, 0, 0, 0, 0, 0]  block_version=10.1.3
  add3_1 : xbsIndex_r4/AddSub  mode=Addition  use_carryin=off  use_carryout=off  en=off  latency=1  precision=Full  arith_type=Unsigned  n_bits=8  bin_pt=2  quantization=Truncate  overflow=Wrap  dbl_ovrd=off  use_behavioral_HDL=on  pipelined=on  use_rpm=on  hw_selection=Fabric  block_type=addsub
      settings: xl_area=[0, 0, 0, 0, 0, 0, 0]  block_version=10.1.3
  mult{1..8} (8 alike) : quant_xcorr_lib/2bit_mult  SystemSampleTime=-1
  acc_out : Outport  OutDataType=sfix(16)  OutScaling=2^0
  wires:
  in1_0/1 -> mult1/1
  in2_0/1 -> mult1/2
  in1_1/1 -> mult2/1
  in2_1/1 -> mult2/2
  in1_2/1 -> mult3/1
  in2_2/1 -> mult3/2
  in1_3/1 -> mult4/1
  in2_3/1 -> mult4/2
  in1_4/1 -> mult5/1
  in2_4/1 -> mult5/2
  in1_5/1 -> mult6/1
  in2_5/1 -> mult6/2
  in1_6/1 -> mult7/1
  in2_6/1 -> mult7/2
  in1_7/1 -> mult8/1
  in2_7/1 -> mult8/2
  mult1/1 -> add1_1/1
  mult2/1 -> add1_1/2
  mult3/1 -> add1_2/1
  mult4/1 -> add1_2/2
  mult5/1 -> add1_3/1
  mult6/1 -> add1_3/2
  mult7/1 -> add1_4/1
  mult8/1 -> add1_4/2
  add1_1/1 -> add2_1/1
  add1_2/1 -> add2_1/2
  add1_3/1 -> add2_2/1
  add1_4/1 -> add2_2/2
  add2_1/1 -> add3_1/1
  add2_2/1 -> add3_1/2
  add3_1/1 -> accumul10/1
  accumul10/1 -> acc_out/1
  rst/1 -> accumul10/2
  en/1 -> accumul10/3

== system bee2_calib_corr/quant_cross_corr1/lag11 ==
  in1_0 : Inport  OutDataType=sfix(16)  OutScaling=2^0
  in1_1 : Inport  Port=2  OutDataType=sfix(16)  OutScaling=2^0
  in1_2 : Inport  Port=3  OutDataType=sfix(16)  OutScaling=2^0
  in1_3 : Inport  Port=4  OutDataType=sfix(16)  OutScaling=2^0
  in1_4 : Inport  Port=5  OutDataType=sfix(16)  OutScaling=2^0
  in1_5 : Inport  Port=6  OutDataType=sfix(16)  OutScaling=2^0
  in1_6 : Inport  Port=7  OutDataType=sfix(16)  OutScaling=2^0
  in1_7 : Inport  Port=8  OutDataType=sfix(16)  OutScaling=2^0
  in2_0 : Inport  Port=9  OutDataType=sfix(16)  OutScaling=2^0
  in2_1 : Inport  Port=10  OutDataType=sfix(16)  OutScaling=2^0
  in2_2 : Inport  Port=11  OutDataType=sfix(16)  OutScaling=2^0
  in2_3 : Inport  Port=12  OutDataType=sfix(16)  OutScaling=2^0
  in2_4 : Inport  Port=13  OutDataType=sfix(16)  OutScaling=2^0
  in2_5 : Inport  Port=14  OutDataType=sfix(16)  OutScaling=2^0
  in2_6 : Inport  Port=15  OutDataType=sfix(16)  OutScaling=2^0
  in2_7 : Inport  Port=16  OutDataType=sfix(16)  OutScaling=2^0
  rst : Inport  Port=17  OutDataType=sfix(16)  OutScaling=2^0
  en : Inport  Port=18  OutDataType=sfix(16)  OutScaling=2^0
  accumul11 : xbsIndex_r4/Accumulator  operation=Add  n_bits=32  overflow=Saturate  scale=1  rst=on  hasbypass=off  en=on  dbl_ovrd=off  use_behavioral_HDL=on  implementation=Fabric  block_type=accum
      settings: infoedit="Adder or subtractor-based accumulator.   Output type and binary point position match the input.<P><P>Hardware notes: When \"Reinitialize with input 'b' on reset\" is selected, the accumulator is forced to run at the system rate even if the input 'b' is running at a slower rate."  xl_area=[0, 0, 0, 0, 0, 0, 0]  block_version=10.1.3
  add1_{1..4} (4 alike) : xbsIndex_r4/AddSub  mode=Addition  use_carryin=off  use_carryout=off  en=off  latency=1  precision=Full  arith_type=Unsigned  n_bits=8  bin_pt=2  quantization=Truncate  overflow=Wrap  dbl_ovrd=off  use_behavioral_HDL=on  pipelined=on  use_rpm=on  hw_selection=Fabric  block_type=addsub
      settings: xl_area=[0, 0, 0, 0, 0, 0, 0]  block_version=10.1.3
  add2_1 : xbsIndex_r4/AddSub  mode=Addition  use_carryin=off  use_carryout=off  en=off  latency=1  precision=Full  arith_type=Unsigned  n_bits=8  bin_pt=2  quantization=Truncate  overflow=Wrap  dbl_ovrd=off  use_behavioral_HDL=on  pipelined=on  use_rpm=on  hw_selection=Fabric  block_type=addsub
      settings: xl_area=[0, 0, 0, 0, 0, 0, 0]  block_version=10.1.3
  add2_2 : xbsIndex_r4/AddSub  mode=Addition  use_carryin=off  use_carryout=off  en=off  latency=1  precision=Full  arith_type=Unsigned  n_bits=8  bin_pt=2  quantization=Truncate  overflow=Wrap  dbl_ovrd=off  use_behavioral_HDL=on  pipelined=on  use_rpm=on  hw_selection=Fabric  block_type=addsub
      settings: xl_area=[0, 0, 0, 0, 0, 0, 0]  block_version=10.1.3
  add3_1 : xbsIndex_r4/AddSub  mode=Addition  use_carryin=off  use_carryout=off  en=off  latency=1  precision=Full  arith_type=Unsigned  n_bits=8  bin_pt=2  quantization=Truncate  overflow=Wrap  dbl_ovrd=off  use_behavioral_HDL=on  pipelined=on  use_rpm=on  hw_selection=Fabric  block_type=addsub
      settings: xl_area=[0, 0, 0, 0, 0, 0, 0]  block_version=10.1.3
  mult{1..8} (8 alike) : quant_xcorr_lib/2bit_mult  SystemSampleTime=-1
  acc_out : Outport  OutDataType=sfix(16)  OutScaling=2^0
  wires:
  in1_0/1 -> mult1/1
  in2_0/1 -> mult1/2
  in1_1/1 -> mult2/1
  in2_1/1 -> mult2/2
  in1_2/1 -> mult3/1
  in2_2/1 -> mult3/2
  in1_3/1 -> mult4/1
  in2_3/1 -> mult4/2
  in1_4/1 -> mult5/1
  in2_4/1 -> mult5/2
  in1_5/1 -> mult6/1
  in2_5/1 -> mult6/2
  in1_6/1 -> mult7/1
  in2_6/1 -> mult7/2
  in1_7/1 -> mult8/1
  in2_7/1 -> mult8/2
  mult1/1 -> add1_1/1
  mult2/1 -> add1_1/2
  mult3/1 -> add1_2/1
  mult4/1 -> add1_2/2
  mult5/1 -> add1_3/1
  mult6/1 -> add1_3/2
  mult7/1 -> add1_4/1
  mult8/1 -> add1_4/2
  add1_1/1 -> add2_1/1
  add1_2/1 -> add2_1/2
  add1_3/1 -> add2_2/1
  add1_4/1 -> add2_2/2
  add2_1/1 -> add3_1/1
  add2_2/1 -> add3_1/2
  add3_1/1 -> accumul11/1
  accumul11/1 -> acc_out/1
  rst/1 -> accumul11/2
  en/1 -> accumul11/3

== system bee2_calib_corr/quant_cross_corr1/lag12 ==
  in1_0 : Inport  OutDataType=sfix(16)  OutScaling=2^0
  in1_1 : Inport  Port=2  OutDataType=sfix(16)  OutScaling=2^0
  in1_2 : Inport  Port=3  OutDataType=sfix(16)  OutScaling=2^0
  in1_3 : Inport  Port=4  OutDataType=sfix(16)  OutScaling=2^0
  in1_4 : Inport  Port=5  OutDataType=sfix(16)  OutScaling=2^0
  in1_5 : Inport  Port=6  OutDataType=sfix(16)  OutScaling=2^0
  in1_6 : Inport  Port=7  OutDataType=sfix(16)  OutScaling=2^0
  in1_7 : Inport  Port=8  OutDataType=sfix(16)  OutScaling=2^0
  in2_0 : Inport  Port=9  OutDataType=sfix(16)  OutScaling=2^0
  in2_1 : Inport  Port=10  OutDataType=sfix(16)  OutScaling=2^0
  in2_2 : Inport  Port=11  OutDataType=sfix(16)  OutScaling=2^0
  in2_3 : Inport  Port=12  OutDataType=sfix(16)  OutScaling=2^0
  in2_4 : Inport  Port=13  OutDataType=sfix(16)  OutScaling=2^0
  in2_5 : Inport  Port=14  OutDataType=sfix(16)  OutScaling=2^0
  in2_6 : Inport  Port=15  OutDataType=sfix(16)  OutScaling=2^0
  in2_7 : Inport  Port=16  OutDataType=sfix(16)  OutScaling=2^0
  rst : Inport  Port=17  OutDataType=sfix(16)  OutScaling=2^0
  en : Inport  Port=18  OutDataType=sfix(16)  OutScaling=2^0
  accumul12 : xbsIndex_r4/Accumulator  operation=Add  n_bits=32  overflow=Saturate  scale=1  rst=on  hasbypass=off  en=on  dbl_ovrd=off  use_behavioral_HDL=on  implementation=Fabric  block_type=accum
      settings: infoedit="Adder or subtractor-based accumulator.   Output type and binary point position match the input.<P><P>Hardware notes: When \"Reinitialize with input 'b' on reset\" is selected, the accumulator is forced to run at the system rate even if the input 'b' is running at a slower rate."  xl_area=[0, 0, 0, 0, 0, 0, 0]  block_version=10.1.3
  add1_{1..4} (4 alike) : xbsIndex_r4/AddSub  mode=Addition  use_carryin=off  use_carryout=off  en=off  latency=1  precision=Full  arith_type=Unsigned  n_bits=8  bin_pt=2  quantization=Truncate  overflow=Wrap  dbl_ovrd=off  use_behavioral_HDL=on  pipelined=on  use_rpm=on  hw_selection=Fabric  block_type=addsub
      settings: xl_area=[0, 0, 0, 0, 0, 0, 0]  block_version=10.1.3
  add2_1 : xbsIndex_r4/AddSub  mode=Addition  use_carryin=off  use_carryout=off  en=off  latency=1  precision=Full  arith_type=Unsigned  n_bits=8  bin_pt=2  quantization=Truncate  overflow=Wrap  dbl_ovrd=off  use_behavioral_HDL=on  pipelined=on  use_rpm=on  hw_selection=Fabric  block_type=addsub
      settings: xl_area=[0, 0, 0, 0, 0, 0, 0]  block_version=10.1.3
  add2_2 : xbsIndex_r4/AddSub  mode=Addition  use_carryin=off  use_carryout=off  en=off  latency=1  precision=Full  arith_type=Unsigned  n_bits=8  bin_pt=2  quantization=Truncate  overflow=Wrap  dbl_ovrd=off  use_behavioral_HDL=on  pipelined=on  use_rpm=on  hw_selection=Fabric  block_type=addsub
      settings: xl_area=[0, 0, 0, 0, 0, 0, 0]  block_version=10.1.3
  add3_1 : xbsIndex_r4/AddSub  mode=Addition  use_carryin=off  use_carryout=off  en=off  latency=1  precision=Full  arith_type=Unsigned  n_bits=8  bin_pt=2  quantization=Truncate  overflow=Wrap  dbl_ovrd=off  use_behavioral_HDL=on  pipelined=on  use_rpm=on  hw_selection=Fabric  block_type=addsub
      settings: xl_area=[0, 0, 0, 0, 0, 0, 0]  block_version=10.1.3
  mult{1..8} (8 alike) : quant_xcorr_lib/2bit_mult  SystemSampleTime=-1
  acc_out : Outport  OutDataType=sfix(16)  OutScaling=2^0
  wires:
  in1_0/1 -> mult1/1
  in2_0/1 -> mult1/2
  in1_1/1 -> mult2/1
  in2_1/1 -> mult2/2
  in1_2/1 -> mult3/1
  in2_2/1 -> mult3/2
  in1_3/1 -> mult4/1
  in2_3/1 -> mult4/2
  in1_4/1 -> mult5/1
  in2_4/1 -> mult5/2
  in1_5/1 -> mult6/1
  in2_5/1 -> mult6/2
  in1_6/1 -> mult7/1
  in2_6/1 -> mult7/2
  in1_7/1 -> mult8/1
  in2_7/1 -> mult8/2
  mult1/1 -> add1_1/1
  mult2/1 -> add1_1/2
  mult3/1 -> add1_2/1
  mult4/1 -> add1_2/2
  mult5/1 -> add1_3/1
  mult6/1 -> add1_3/2
  mult7/1 -> add1_4/1
  mult8/1 -> add1_4/2
  add1_1/1 -> add2_1/1
  add1_2/1 -> add2_1/2
  add1_3/1 -> add2_2/1
  add1_4/1 -> add2_2/2
  add2_1/1 -> add3_1/1
  add2_2/1 -> add3_1/2
  add3_1/1 -> accumul12/1
  accumul12/1 -> acc_out/1
  rst/1 -> accumul12/2
  en/1 -> accumul12/3

== system bee2_calib_corr/quant_cross_corr1/lag13 ==
  in1_0 : Inport  OutDataType=sfix(16)  OutScaling=2^0
  in1_1 : Inport  Port=2  OutDataType=sfix(16)  OutScaling=2^0
  in1_2 : Inport  Port=3  OutDataType=sfix(16)  OutScaling=2^0
  in1_3 : Inport  Port=4  OutDataType=sfix(16)  OutScaling=2^0
  in1_4 : Inport  Port=5  OutDataType=sfix(16)  OutScaling=2^0
  in1_5 : Inport  Port=6  OutDataType=sfix(16)  OutScaling=2^0
  in1_6 : Inport  Port=7  OutDataType=sfix(16)  OutScaling=2^0
  in1_7 : Inport  Port=8  OutDataType=sfix(16)  OutScaling=2^0
  in2_0 : Inport  Port=9  OutDataType=sfix(16)  OutScaling=2^0
  in2_1 : Inport  Port=10  OutDataType=sfix(16)  OutScaling=2^0
  in2_2 : Inport  Port=11  OutDataType=sfix(16)  OutScaling=2^0
  in2_3 : Inport  Port=12  OutDataType=sfix(16)  OutScaling=2^0
  in2_4 : Inport  Port=13  OutDataType=sfix(16)  OutScaling=2^0
  in2_5 : Inport  Port=14  OutDataType=sfix(16)  OutScaling=2^0
  in2_6 : Inport  Port=15  OutDataType=sfix(16)  OutScaling=2^0
  in2_7 : Inport  Port=16  OutDataType=sfix(16)  OutScaling=2^0
  rst : Inport  Port=17  OutDataType=sfix(16)  OutScaling=2^0
  en : Inport  Port=18  OutDataType=sfix(16)  OutScaling=2^0
  accumul13 : xbsIndex_r4/Accumulator  operation=Add  n_bits=32  overflow=Saturate  scale=1  rst=on  hasbypass=off  en=on  dbl_ovrd=off  use_behavioral_HDL=on  implementation=Fabric  block_type=accum
      settings: infoedit="Adder or subtractor-based accumulator.   Output type and binary point position match the input.<P><P>Hardware notes: When \"Reinitialize with input 'b' on reset\" is selected, the accumulator is forced to run at the system rate even if the input 'b' is running at a slower rate."  xl_area=[0, 0, 0, 0, 0, 0, 0]  block_version=10.1.3
  add1_{1..4} (4 alike) : xbsIndex_r4/AddSub  mode=Addition  use_carryin=off  use_carryout=off  en=off  latency=1  precision=Full  arith_type=Unsigned  n_bits=8  bin_pt=2  quantization=Truncate  overflow=Wrap  dbl_ovrd=off  use_behavioral_HDL=on  pipelined=on  use_rpm=on  hw_selection=Fabric  block_type=addsub
      settings: xl_area=[0, 0, 0, 0, 0, 0, 0]  block_version=10.1.3
  add2_1 : xbsIndex_r4/AddSub  mode=Addition  use_carryin=off  use_carryout=off  en=off  latency=1  precision=Full  arith_type=Unsigned  n_bits=8  bin_pt=2  quantization=Truncate  overflow=Wrap  dbl_ovrd=off  use_behavioral_HDL=on  pipelined=on  use_rpm=on  hw_selection=Fabric  block_type=addsub
      settings: xl_area=[0, 0, 0, 0, 0, 0, 0]  block_version=10.1.3
  add2_2 : xbsIndex_r4/AddSub  mode=Addition  use_carryin=off  use_carryout=off  en=off  latency=1  precision=Full  arith_type=Unsigned  n_bits=8  bin_pt=2  quantization=Truncate  overflow=Wrap  dbl_ovrd=off  use_behavioral_HDL=on  pipelined=on  use_rpm=on  hw_selection=Fabric  block_type=addsub
      settings: xl_area=[0, 0, 0, 0, 0, 0, 0]  block_version=10.1.3
  add3_1 : xbsIndex_r4/AddSub  mode=Addition  use_carryin=off  use_carryout=off  en=off  latency=1  precision=Full  arith_type=Unsigned  n_bits=8  bin_pt=2  quantization=Truncate  overflow=Wrap  dbl_ovrd=off  use_behavioral_HDL=on  pipelined=on  use_rpm=on  hw_selection=Fabric  block_type=addsub
      settings: xl_area=[0, 0, 0, 0, 0, 0, 0]  block_version=10.1.3
  mult{1..8} (8 alike) : quant_xcorr_lib/2bit_mult  SystemSampleTime=-1
  acc_out : Outport  OutDataType=sfix(16)  OutScaling=2^0
  wires:
  in1_0/1 -> mult1/1
  in2_0/1 -> mult1/2
  in1_1/1 -> mult2/1
  in2_1/1 -> mult2/2
  in1_2/1 -> mult3/1
  in2_2/1 -> mult3/2
  in1_3/1 -> mult4/1
  in2_3/1 -> mult4/2
  in1_4/1 -> mult5/1
  in2_4/1 -> mult5/2
  in1_5/1 -> mult6/1
  in2_5/1 -> mult6/2
  in1_6/1 -> mult7/1
  in2_6/1 -> mult7/2
  in1_7/1 -> mult8/1
  in2_7/1 -> mult8/2
  mult1/1 -> add1_1/1
  mult2/1 -> add1_1/2
  mult3/1 -> add1_2/1
  mult4/1 -> add1_2/2
  mult5/1 -> add1_3/1
  mult6/1 -> add1_3/2
  mult7/1 -> add1_4/1
  mult8/1 -> add1_4/2
  add1_1/1 -> add2_1/1
  add1_2/1 -> add2_1/2
  add1_3/1 -> add2_2/1
  add1_4/1 -> add2_2/2
  add2_1/1 -> add3_1/1
  add2_2/1 -> add3_1/2
  add3_1/1 -> accumul13/1
  accumul13/1 -> acc_out/1
  rst/1 -> accumul13/2
  en/1 -> accumul13/3
